simulink model "hyd_hyb_2_opt_cont_test_new_1_col_cd"  (Simulink 7.7)
settings: Solver=ode14x  StartTime=0  StopTime=640  FixedStep=0.01  MaxStep=.4  RelTol=1e-8
InitFcn:
  | const_3
values from neighbouring files (symbols this model uses):
  Af = 6.3    [brk_enrg.m]    (also in brk_enrg_1.m)
  BSFC_906_205 = [3.5684 4.3466 5.1668 6.0769 6.988 7.8992 8.8104 9.7336 10.8548 11.9809 13.1112 14.2571 ... (663 elements, 39x17)]    [BSFC_map_906_205.mat]
  Cd = 0.55    [brk_enrg.m]    (also in brk_enrg_1.m)
  den = 1.202    [brk_enrg.m]    (also in brk_enrg_1.m)
  fr = 0.01    [brk_enrg.m]    (also in brk_enrg_1.m)
undefined symbols (not defined in the file or in its folder): clutchRise, down_spd_2, down_spd_3, down_spd_4, down_spd_c, fuel_density, fuz_wgt, gearshift, I_wheel, Idle_spd_eng_906, M1_hydr, p_v_15, pmax_125, qv_125, Rw, sec_eng_sfc_map, T_max_906, T_min, TC_spd_ratio_max, torque_convertor, Twait, up_spd_1, up_spd_2, up_spd_3, up_spd_c, v_p_10, v_p_15, v_p_5
libraries: neural x4605, fl_lib x43, nesl_utility x30, sdl_lib x14, simulink x4, simulink_need_slupdate x1
(part 114 of 114)

== matlab function controller1/optimized controller - pm1/SOC upper 0.5/SOC upper 0.6/SOC upper 0.7/SOC under 0.8/Subsystem1/MATLAB Function ==
  data: t input double; y output double; fuz_wgt input double
function y = fcn(t,fuz_wgt)
if t<=100
    y=fuz_wgt(1,1);
elseif t<=200
    y=fuz_wgt(1,2);
elseif t<=300
    y=fuz_wgt(1,3);
elseif t<=400
    y=fuz_wgt(1,4);
elseif t<=500
    y=fuz_wgt(1,5);
else
    y=fuz_wgt(1,6);
end

== matlab function controller1/optimized controller - pm1/SOC upper 0.5/SOC upper 0.6/SOC upper 0.7/SOC under 0.8/Subsystem2/MATLAB Function ==
  data: t input double; y output double; fuz_wgt input double
function y = fcn(t,fuz_wgt)
if t<=100
    y=fuz_wgt(2,1);
elseif t<=200
    y=fuz_wgt(2,2);
elseif t<=300
    y=fuz_wgt(2,3);
elseif t<=400
    y=fuz_wgt(2,4);
elseif t<=500
    y=fuz_wgt(2,5);
else
    y=fuz_wgt(2,6);
end

== matlab function controller1/optimized controller - pm1/SOC upper 0.5/SOC upper 0.6/SOC upper 0.7/SOC under 0.8/Subsystem3/MATLAB Function ==
  data: t input double; y output double; fuz_wgt input double
function y = fcn(t,fuz_wgt)
if t<=100
    y=fuz_wgt(3,1);
elseif t<=200
    y=fuz_wgt(3,2);
elseif t<=300
    y=fuz_wgt(3,3);
elseif t<=400
    y=fuz_wgt(3,4);
elseif t<=500
    y=fuz_wgt(3,5);
else
    y=fuz_wgt(3,6);
end

== matlab function controller1/optimized controller - pm1/SOC upper 0.5/SOC upper 0.6/SOC upper 0.7/SOC upper 0.8/Subsystem/MATLAB Function ==
  data: t input double; y output double; fuz_wgt input double
function y = fcn(t,fuz_wgt)
if t<=100
    y=fuz_wgt(4,1);
elseif t<=200
    y=fuz_wgt(4,2);
elseif t<=300
    y=fuz_wgt(4,3);
elseif t<=400
    y=fuz_wgt(4,4);
elseif t<=500
    y=fuz_wgt(4,5);
else
    y=fuz_wgt(4,6);
end

== matlab function controller1/optimized controller - pm1/SOC upper 0.5/SOC upper 0.6/SOC upper 0.7/SOC upper 0.8/Subsystem1/MATLAB Function ==
  data: t input double; y output double; fuz_wgt input double
function y = fcn(t,fuz_wgt)
if t<=100
    y=fuz_wgt(1,1);
elseif t<=200
    y=fuz_wgt(1,2);
elseif t<=300
    y=fuz_wgt(1,3);
elseif t<=400
    y=fuz_wgt(1,4);
elseif t<=500
    y=fuz_wgt(1,5);
else
    y=fuz_wgt(1,6);
end

== matlab function controller1/optimized controller - pm1/SOC upper 0.5/SOC upper 0.6/SOC upper 0.7/SOC upper 0.8/Subsystem2/MATLAB Function ==
  data: t input double; y output double; fuz_wgt input double
function y = fcn(t,fuz_wgt)
if t<=100
    y=fuz_wgt(2,1);
elseif t<=200
    y=fuz_wgt(2,2);
elseif t<=300
    y=fuz_wgt(2,3);
elseif t<=400
    y=fuz_wgt(2,4);
elseif t<=500
    y=fuz_wgt(2,5);
else
    y=fuz_wgt(2,6);
end

== matlab function controller1/optimized controller - pm1/SOC upper 0.5/SOC upper 0.6/SOC upper 0.7/SOC upper 0.8/Subsystem3/MATLAB Function ==
  data: t input double; y output double; fuz_wgt input double
function y = fcn(t,fuz_wgt)
if t<=100
    y=fuz_wgt(3,1);
elseif t<=200
    y=fuz_wgt(3,2);
elseif t<=300
    y=fuz_wgt(3,3);
elseif t<=400
    y=fuz_wgt(3,4);
elseif t<=500
    y=fuz_wgt(3,5);
else
    y=fuz_wgt(3,6);
end

== matlab function controller1/optimized controller - pm/SOC under 0.5/Subsystem/MATLAB Function ==
  data: t input double; y output double; fuz_wgt input double
function y = fcn(t,fuz_wgt)
if t<=100
    y=fuz_wgt(4,1);
elseif t<=200
    y=fuz_wgt(4,2);
elseif t<=300
    y=fuz_wgt(4,3);
elseif t<=400
    y=fuz_wgt(4,4);
elseif t<=500
    y=fuz_wgt(4,5);
else
    y=fuz_wgt(4,6);
end
end

== matlab function controller1/optimized controller - pm/SOC under 0.5/Subsystem1/MATLAB Function ==
  data: t input double; y output double; fuz_wgt input double
function y = fcn(t,fuz_wgt)
if t<=100
    y=fuz_wgt(1,1);
elseif t<=200
    y=fuz_wgt(1,2);
elseif t<=300
    y=fuz_wgt(1,3);
elseif t<=400
    y=fuz_wgt(1,4);
elseif t<=500
    y=fuz_wgt(1,5);
else
    y=fuz_wgt(1,6);
end

== matlab function controller1/optimized controller - pm/SOC under 0.5/Subsystem2/MATLAB Function ==
  data: t input double; y output double; fuz_wgt input double
function y = fcn(t,fuz_wgt)
if t<=100
    y=fuz_wgt(2,1);
elseif t<=200
    y=fuz_wgt(2,2);
elseif t<=300
    y=fuz_wgt(2,3);
elseif t<=400
    y=fuz_wgt(2,4);
elseif t<=500
    y=fuz_wgt(2,5);
else
    y=fuz_wgt(2,6);
end

== matlab function controller1/optimized controller - pm/SOC under 0.5/Subsystem3/MATLAB Function ==
  data: t input double; y output double; fuz_wgt input double
function y = fcn(t,fuz_wgt)
if t<=100
    y=fuz_wgt(3,1);
elseif t<=200
    y=fuz_wgt(3,2);
elseif t<=300
    y=fuz_wgt(3,3);
elseif t<=400
    y=fuz_wgt(3,4);
elseif t<=500
    y=fuz_wgt(3,5);
else
    y=fuz_wgt(3,6);
end

== matlab function controller1/optimized controller - pm/SOC under 0.5/col_c_d1/MATLAB Function ==
  data: soc input double; u_0_4 output double; u_4_5 output double
function [u_0_4,u_4_5] = fcn(soc)
if soc<0.37
    u_0_4=1;
    u_4_5=0;
elseif soc<0.42
    u_0_4=(-1/0.05)*(soc-.42);
    u_4_5=(1/0.05)*(soc-.37);
else
    u_0_4=0;
    u_4_5=1;
end
end

== matlab function controller1/optimized controller - pm/SOC upper 0.5/SOC under 0.6/fuz_wgt_col_a_b/MATLAB Function ==
  data: t input double; y output double; fuz_wgt input double
function y = fcn(t,fuz_wgt)
if t<=100
    y=fuz_wgt(1,1);
elseif t<=200
    y=fuz_wgt(1,2);
elseif t<=300
    y=fuz_wgt(1,3);
elseif t<=400
    y=fuz_wgt(1,4);
elseif t<=500
    y=fuz_wgt(1,5);
else
    y=fuz_wgt(1,6);
end

== matlab function controller1/optimized controller - pm/SOC upper 0.5/SOC under 0.6/fuz_wgt_col_c_d/MATLAB Function ==
  data: t input double; y output double; fuz_wgt input double
function y = fcn(t,fuz_wgt)
if t<=100
    y=fuz_wgt(2,1);
elseif t<=200
    y=fuz_wgt(2,2);
elseif t<=300
    y=fuz_wgt(2,3);
elseif t<=400
    y=fuz_wgt(2,4);
elseif t<=500
    y=fuz_wgt(2,5);
else
    y=fuz_wgt(2,6);
end

== matlab function controller1/optimized controller - pm/SOC upper 0.5/SOC under 0.6/fuz_wgt_col_e_f/MATLAB Function ==
  data: t input double; y output double; fuz_wgt input double
function y = fcn(t,fuz_wgt)
if t<=100
    y=fuz_wgt(3,1);
elseif t<=200
    y=fuz_wgt(3,2);
elseif t<=300
    y=fuz_wgt(3,3);
elseif t<=400
    y=fuz_wgt(3,4);
elseif t<=500
    y=fuz_wgt(3,5);
else
    y=fuz_wgt(3,6);
end

== matlab function controller1/optimized controller - pm/SOC upper 0.5/SOC under 0.6/fuz_wgt_loc_rod/MATLAB Function ==
  data: t input double; y output double; fuz_wgt input double
function y = fcn(t,fuz_wgt)
if t<=100
    y=fuz_wgt(4,1);
elseif t<=200
    y=fuz_wgt(4,2);
elseif t<=300
    y=fuz_wgt(4,3);
elseif t<=400
    y=fuz_wgt(4,4);
elseif t<=500
    y=fuz_wgt(4,5);
else
    y=fuz_wgt(4,6);
end

== matlab function controller1/optimized controller - pm/SOC upper 0.5/SOC upper 0.6/SOC under 0.7/Subsystem/MATLAB Function ==
  data: t input double; y output double; fuz_wgt input double
function y = fcn(t,fuz_wgt)
if t<=100
    y=fuz_wgt(4,1);
elseif t<=200
    y=fuz_wgt(4,2);
elseif t<=300
    y=fuz_wgt(4,3);
elseif t<=400
    y=fuz_wgt(4,4);
elseif t<=500
    y=fuz_wgt(4,5);
else
    y=fuz_wgt(4,6);
end

== matlab function controller1/optimized controller - pm/SOC upper 0.5/SOC upper 0.6/SOC under 0.7/Subsystem1/MATLAB Function ==
  data: t input double; y output double; fuz_wgt input double
function y = fcn(t,fuz_wgt)
if t<=100
    y=fuz_wgt(1,1);
elseif t<=200
    y=fuz_wgt(1,2);
elseif t<=300
    y=fuz_wgt(1,3);
elseif t<=400
    y=fuz_wgt(1,4);
elseif t<=500
    y=fuz_wgt(1,5);
else
    y=fuz_wgt(1,6);
end

== matlab function controller1/optimized controller - pm/SOC upper 0.5/SOC upper 0.6/SOC under 0.7/Subsystem2/MATLAB Function ==
  data: t input double; y output double; fuz_wgt input double
function y = fcn(t,fuz_wgt)
if t<=100
    y=fuz_wgt(2,1);
elseif t<=200
    y=fuz_wgt(2,2);
elseif t<=300
    y=fuz_wgt(2,3);
elseif t<=400
    y=fuz_wgt(2,4);
elseif t<=500
    y=fuz_wgt(2,5);
else
    y=fuz_wgt(2,6);
end

== matlab function controller1/optimized controller - pm/SOC upper 0.5/SOC upper 0.6/SOC under 0.7/Subsystem3/MATLAB Function ==
  data: t input double; y output double; fuz_wgt input double
function y = fcn(t,fuz_wgt)
if t<=100
    y=fuz_wgt(3,1);
elseif t<=200
    y=fuz_wgt(3,2);
elseif t<=300
    y=fuz_wgt(3,3);
elseif t<=400
    y=fuz_wgt(3,4);
elseif t<=500
    y=fuz_wgt(3,5);
else
    y=fuz_wgt(3,6);
end

== matlab function controller1/optimized controller - pm/SOC upper 0.5/SOC upper 0.6/SOC upper 0.7/SOC under 0.8/Subsystem/MATLAB Function ==
  data: t input double; y output double; fuz_wgt input double
function y = fcn(t,fuz_wgt)
if t<=100
    y=fuz_wgt(4,1);
elseif t<=200
    y=fuz_wgt(4,2);
elseif t<=300
    y=fuz_wgt(4,3);
elseif t<=400
    y=fuz_wgt(4,4);
elseif t<=500
    y=fuz_wgt(4,5);
else
    y=fuz_wgt(4,6);
end

== matlab function controller1/optimized controller - pm/SOC upper 0.5/SOC upper 0.6/SOC upper 0.7/SOC under 0.8/Subsystem1/MATLAB Function ==
  data: t input double; y output double; fuz_wgt input double
function y = fcn(t,fuz_wgt)
if t<=100
    y=fuz_wgt(1,1);
elseif t<=200
    y=fuz_wgt(1,2);
elseif t<=300
    y=fuz_wgt(1,3);
elseif t<=400
    y=fuz_wgt(1,4);
elseif t<=500
    y=fuz_wgt(1,5);
else
    y=fuz_wgt(1,6);
end

== matlab function controller1/optimized controller - pm/SOC upper 0.5/SOC upper 0.6/SOC upper 0.7/SOC under 0.8/Subsystem2/MATLAB Function ==
  data: t input double; y output double; fuz_wgt input double
function y = fcn(t,fuz_wgt)
if t<=100
    y=fuz_wgt(2,1);
elseif t<=200
    y=fuz_wgt(2,2);
elseif t<=300
    y=fuz_wgt(2,3);
elseif t<=400
    y=fuz_wgt(2,4);
elseif t<=500
    y=fuz_wgt(2,5);
else
    y=fuz_wgt(2,6);
end

== matlab function controller1/optimized controller - pm/SOC upper 0.5/SOC upper 0.6/SOC upper 0.7/SOC under 0.8/Subsystem3/MATLAB Function ==
  data: t input double; y output double; fuz_wgt input double
function y = fcn(t,fuz_wgt)
if t<=100
    y=fuz_wgt(3,1);
elseif t<=200
    y=fuz_wgt(3,2);
elseif t<=300
    y=fuz_wgt(3,3);
elseif t<=400
    y=fuz_wgt(3,4);
elseif t<=500
    y=fuz_wgt(3,5);
else
    y=fuz_wgt(3,6);
end

== matlab function controller1/optimized controller - pm/SOC upper 0.5/SOC upper 0.6/SOC upper 0.7/SOC upper 0.8/Subsystem/MATLAB Function ==
  data: t input double; y output double; fuz_wgt input double
function y = fcn(t,fuz_wgt)
if t<=100
    y=fuz_wgt(4,1);
elseif t<=200
    y=fuz_wgt(4,2);
elseif t<=300
    y=fuz_wgt(4,3);
elseif t<=400
    y=fuz_wgt(4,4);
elseif t<=500
    y=fuz_wgt(4,5);
else
    y=fuz_wgt(4,6);
end

== matlab function controller1/optimized controller - pm/SOC upper 0.5/SOC upper 0.6/SOC upper 0.7/SOC upper 0.8/Subsystem1/MATLAB Function ==
  data: t input double; y output double; fuz_wgt input double
function y = fcn(t,fuz_wgt)
if t<=100
    y=fuz_wgt(1,1);
elseif t<=200
    y=fuz_wgt(1,2);
elseif t<=300
    y=fuz_wgt(1,3);
elseif t<=400
    y=fuz_wgt(1,4);
elseif t<=500
    y=fuz_wgt(1,5);
else
    y=fuz_wgt(1,6);
end

== matlab function controller1/optimized controller - pm/SOC upper 0.5/SOC upper 0.6/SOC upper 0.7/SOC upper 0.8/Subsystem2/MATLAB Function ==
  data: t input double; y output double; fuz_wgt input double
function y = fcn(t,fuz_wgt)
if t<=100
    y=fuz_wgt(2,1);
elseif t<=200
    y=fuz_wgt(2,2);
elseif t<=300
    y=fuz_wgt(2,3);
elseif t<=400
    y=fuz_wgt(2,4);
elseif t<=500
    y=fuz_wgt(2,5);
else
    y=fuz_wgt(2,6);
end

== matlab function controller1/optimized controller - pm/SOC upper 0.5/SOC upper 0.6/SOC upper 0.7/SOC upper 0.8/Subsystem3/MATLAB Function ==
  data: t input double; y output double; fuz_wgt input double
function y = fcn(t,fuz_wgt)
if t<=100
    y=fuz_wgt(3,1);
elseif t<=200
    y=fuz_wgt(3,2);
elseif t<=300
    y=fuz_wgt(3,3);
elseif t<=400
    y=fuz_wgt(3,4);
elseif t<=500
    y=fuz_wgt(3,5);
else
    y=fuz_wgt(3,6);
end
